simulink model "AcasXuClosedLoop_all_comp"  (Simulink 10.0)
settings: Solver=VariableStepAuto  StartTime=0.0  StopTime=20  RelTol=1e-3
libraries: neural x14040, simulink x2
(part 17 of 534)

== system AcasXuClosedLoop_all_comp/networks/Networks_m_3/AcasXu_5_3/Process Output 1 ==
  a : Inport  PortDimensions=5
      mask Display: e = 0.06;c1 = [120 230 180]/255;c2 = ([120 230 180]+0)/(256+128);c3 = ([120 230 180]+128)/(256+128);patch([0 1 1 0],[0 0 1 1],c1);patch([0 1 1 1-e 1-e 0+e],[0 0 1 1-e 0+e 0+e],c2);patch([0 0 1 1-e 0+e 0+e],[0 1 1 1-e 1-e 0+e],c3);plot([0 1 1 0 0],[0 0 1 1 0]);
  y : Outport  PortDimensions=5
      mask Display: e = 0.06;c1 = [120 230 180]/255;c2 = ([120 230 180]+0)/(256+128);c3 = ([120 230 180]+128)/(256+128);patch([0 1 1 0],[0 0 1 1],c1);patch([0 1 1 1-e 1-e 0+e],[0 0 1 1-e 0+e 0+e],c2);patch([0 0 1 1-e 0+e 0+e],[0 1 1 1-e 1-e 0+e],c3);plot([0 1 1 0 0],[0 0 1 1 0]);
  wires:
  a/1 -> y/1

== system AcasXuClosedLoop_all_comp/networks/Networks_m_4/AcasXu_1_4/Layer 1 ==
  "p{1}" : Inport  PortDimensions=5
      mask Display: e = 0.06;c1 = [120 230 180]/255;c2 = ([120 230 180]+0)/(256+128);c3 = ([120 230 180]+128)/(256+128);patch([0 1 1 0],[0 0 1 1],c1);patch([0 1 1 1-e 1-e 0+e],[0 0 1 1-e 0+e 0+e],c2);patch([0 0 1 1-e 0+e 0+e],[0 1 1 1-e 1-e 0+e],c3);plot([0 1 1 0 0],[0 0 1 1 0]);
  Delays 1 : Subsystem
      mask Display: e = 0.06;c1 = [120 150 230]/255;c2 = ([120 150 230]+0)/383;c3 = ([120 150 230]+128)/383;patch([0 1 1 0],[0 0 1 1],c1);patch([0 1 1 1-e 1-e 0+e],[0 0 1 1-e 0+e 0+e],c2);patch([0 0 1 1-e 0+e 0+e],[0 1 1 1-e 1-e 0+e],c3);plot([0 1 1 0 0],[0 0 1 1 0]);disp('0');
  "IW{1,1}" : Subsystem
      mask Display: e = 0.06;c1 = [120 150 230]/255;c2 = ([120 150 230]+0)/383;c3 = ([120 150 230]+128)/383;patch([0 1 1 0],[0 0 1 1],c1);patch([0 1 1 1-e 1-e 0+e],[0 0 1 1-e 0+e 0+e],c2);patch([0 0 1 1-e 0+e 0+e],[0 1 1 1-e 1-e 0+e],c3);plot([0 1 1 0 0],[0 0 1 1 0]);disp('W');
  "b{1}" : Constant  Value=[-0.369373000000000006881606395836570300161838531494140625;0.0031518499999999998774369291965058437199331820011138916015625;0.003017829999999999991688870437656078138388693332672119140625;0.2210229999999999972448705420902115292847156524658203125;-0.07530870000000000619166939941351301968097686767578125;-0.026249899999999999622435353785476763732731342315673828125;-0.34564899999999998403410472747054882347583770751953125;-0.1547409999999999896669322652087430469691753387451171875;-0.273818999999999979078069145543850027024745941162109375;0.17357800000000001006128513836301863193511962890625;-0.265224999999999988542498385868384502828121185302734375;0.000613303000000000046905423989329619871568866074085235595703125;-0.306727000000000027402080604588263668119907379150390625;-0.279696000000000000174082970261224545538425445556640625;0.251846000000000014296119843493215739727020263671875;0.09491080000000000349569262425575288943946361541748046875;0.07782429999999999903348424368232372216880321502685546875;-0.16648899999999999810285089552053250372409820556640625;-0.351362000000000007648992550457478500902652740478515625;-0.004800220000000000007689404668553834198974072933197021484375;0.115561999999999998056665617696125991642475128173828125;-0.255651000000000017120527218139613978564739227294921875;-0.10977699999999999957989160748184076510369777679443359375;-0.1637280000000000124149579505683504976332187652587890625;0.14482600000000001028155338644864968955516815185546875;-0.1920590000000000074908967917508562095463275909423828125;0.083848599999999995358024307279265485703945159912109375;0.041894300000000002370814655705544282682240009307861328125;-0.2147469999999999934470196194524760358035564422607421875;0.07221950000000000591260373994373367168009281158447265625;0.053900999999999997525090833505601040087640285491943359375;0.0011389699999999999997790656180995938484556972980499267578125;-0.17640800000000000924416099223890341818332672119140625;-0.0249854000000000013581580304844464990310370922088623046875;-0.281220999999999998752997498741024173796176910400390625;0.11540799999999999669864081397463451139628887176513671875;0.030775799999999998990407590326867648400366306304931640625;0.00082675499999999998122779398812554063624702394008636474609375;-0.09438370000000000092921226269027101807296276092529296875;-0.51336899999999996424548953655175864696502685546875;-0.23788500000000001310951347477384842932224273681640625;0.07809370000000000200390815052742254920303821563720703125;-0.30906000000000000138555833473219536244869232177734375;-0.1812979999999999869420008735687588341534137725830078125;0.16301799999999999624122892782907001674175262451171875;-0.1350470000000000003748112931134528480470180511474609375;0.0954322999999999976861175809972337447106838226318359375;-0.08284819999999999684536788890909519977867603302001953125;-0.1302379999999999926618698964375653304159641265869140625;0.16506399999999998851052396275917999446392059326171875]
      mask Display: e = 0.06;c1 = [120 150 230]/255;c2 = ([120 150 230]+0)/383;c3 = ([120 150 230]+128)/383;patch([0 1 1 0],[0 0 1 1],c1);patch([0 1 1 1-e 1-e 0+e],[0 0 1 1-e 0+e 0+e],c2);patch([0 0 1 1-e 0+e 0+e],[0 1 1 1-e 1-e 0+e],c3);plot([0 1 1 0 0],[0 0 1 1 0]);disp('b');
  netsum : Sum  Inputs=2  SaturateOnIntegerOverflow=on  InputSameDT=on  OutDataTypeStr=Inherit: Same as first input
      mask Display: e = 0.06;c1 = [120 150 230]/255;c2 = ([120 150 230]+0)/383;c3 = ([120 150 230]+128)/383;patch([0 1 1 0],[0 0 1 1],c1);patch([0 1 1 1-e 1-e 0+e],[0 0 1 1-e 0+e 0+e],c2);patch([0 0 1 1-e 0+e 0+e],[0 1 1 1-e 1-e 0+e],c3);plot([0 1 1 0 0],[0 0 1 1 0]);disp('+');
  poslin : neural/Transfer Functions/poslin
      settings: SourceProductName=Deep Learning Toolbox  SourceProductBaseCode=NN
  "a{1}" : Outport  InitialOutput=[0;0;0;0;0;0;0;0;0;0;0;0;0;0;0;0;0;0;0;0;0;0;0;0;0;0;0;0;0;0;0;0;0;0;0;0;0;0;0;0;0;0;0;0;0;0;0;0;0;0]
      mask Display: e = 0.06;c1 = [120 230 180]/255;c2 = ([120 230 180]+0)/(256+128);c3 = ([120 230 180]+128)/(256+128);patch([0 1 1 0],[0 0 1 1],c1);patch([0 1 1 1-e 1-e 0+e],[0 0 1 1-e 0+e 0+e],c2);patch([0 0 1 1-e 0+e 0+e],[0 1 1 1-e 1-e 0+e],c3);plot([0 1 1 0 0],[0 0 1 1 0]);
  wires:
  poslin/1 -> "a{1}"/1
  netsum/1 -> poslin/1
  "b{1}"/1 -> netsum/2
  "IW{1,1}"/1("iz{1,1}") -> netsum/1
  Delays 1/1("pd{1,1}") -> "IW{1,1}"/1("pd{1,1}")
  "p{1}"/1 -> Delays 1/1("p{1}")

== system AcasXuClosedLoop_all_comp/networks/Networks_m_4/AcasXu_1_4/Layer 2 ==
  "a{1} " : Inport  PortDimensions=50
      mask Display: e = 0.06;c1 = [120 230 180]/255;c2 = ([120 230 180]+0)/(256+128);c3 = ([120 230 180]+128)/(256+128);patch([0 1 1 0],[0 0 1 1],c1);patch([0 1 1 1-e 1-e 0+e],[0 0 1 1-e 0+e 0+e],c2);patch([0 0 1 1-e 0+e 0+e],[0 1 1 1-e 1-e 0+e],c3);plot([0 1 1 0 0],[0 0 1 1 0]);
  Delays 1 : Subsystem
      mask Display: e = 0.06;c1 = [120 150 230]/255;c2 = ([120 150 230]+0)/383;c3 = ([120 150 230]+128)/383;patch([0 1 1 0],[0 0 1 1],c1);patch([0 1 1 1-e 1-e 0+e],[0 0 1 1-e 0+e 0+e],c2);patch([0 0 1 1-e 0+e 0+e],[0 1 1 1-e 1-e 0+e],c3);plot([0 1 1 0 0],[0 0 1 1 0]);disp('0');
  "LW{2,1}" : Subsystem
      mask Display: e = 0.06;c1 = [120 150 230]/255;c2 = ([120 150 230]+0)/383;c3 = ([120 150 230]+128)/383;patch([0 1 1 0],[0 0 1 1],c1);patch([0 1 1 1-e 1-e 0+e],[0 0 1 1-e 0+e 0+e],c2);patch([0 0 1 1-e 0+e 0+e],[0 1 1 1-e 1-e 0+e],c3);plot([0 1 1 0 0],[0 0 1 1 0]);disp('W');
  "b{2}" : Constant  Value=[0.3821689999999999809432438269141130149364471435546875;0.001662480000000000034121594438829561113379895687103271484375;-0.137998000000000009546141654936945997178554534912109375;-0.2448539999999999883240064946221536956727504730224609375;-0.325384999999999979802822736019152216613292694091796875;-0.0245271999999999990027532703606993891298770904541015625;-0.1107599999999999973443465250966255553066730499267578125;-0.445537000000000016353141063518705777823925018310546875;-0.0850719000000000058481219866735045798122882843017578125;0.1055770000000000041762149294299888424575328826904296875;-0.4239129999999999842685838302713818848133087158203125;0.115090999999999998859578909105039201676845550537109375;0.0655763000000000040312642113349284045398235321044921875;-0.0162038000000000008415934615868536639027297496795654296875;0.0002406040000000000023301638396588941759546287357807159423828125;0.27527299999999998991739857956417836248874664306640625;0.25459500000000001573852159708621911704540252685546875;-0.0222078999999999991687982259236378013156354427337646484375;-0.01405660000000000069142469527605499024502933025360107421875;-0.014699099999999999666133732034722925163805484771728515625;-0.00874918000000000049831694326485376222990453243255615234375;0.2137320000000000053130833066461491398513317108154296875;0.064714400000000005253042445474420674145221710205078125;-0.034360099999999997588684408356130006723105907440185546875;0.28537000000000001254107928616576828062534332275390625;0.251249000000000000110134124042815528810024261474609375;0.145390999999999992464694287264137528836727142333984375;-0.264349000000000000643041175862890668213367462158203125;0.31018000000000001126210236179758794605731964111328125;0.218685999999999991505461593988002277910709381103515625;-0.1979849999999999943245398981161997653543949127197265625;0.203643999999999991690202705285628326237201690673828125;0.00621389999999999963209429409971562563441693782806396484375;-0.01057770000000000064355187845421824022196233272552490234375;0.25469799999999997996980027892277576029300689697265625;-0.0206958999999999997132515971998145687393844127655029296875;0.1389869999999999994333421682313201017677783966064453125;0.11972199999999999509014969589770771563053131103515625;0.457021999999999983810283765706117264926433563232421875;0.12488100000000000588951110103153041563928127288818359375;-0.004474180000000000344273498598113292246125638484954833984375;-0.22550700000000001299582663705223239958286285400390625;0.61899000000000004018119170723366551101207733154296875;-0.017492000000000000603517236186235095374286174774169921875;-0.08685249999999999914734871708787977695465087890625;0.26463300000000000711253278495860286056995391845703125;-0.0424145999999999967489117125296615995466709136962890625;0.16082099999999999173638798311003483831882476806640625;0.036690300000000002078603955624203081242740154266357421875;-0.06109900000000000053201887340037501417100429534912109375]
      mask Display: e = 0.06;c1 = [120 150 230]/255;c2 = ([120 150 230]+0)/383;c3 = ([120 150 230]+128)/383;patch([0 1 1 0],[0 0 1 1],c1);patch([0 1 1 1-e 1-e 0+e],[0 0 1 1-e 0+e 0+e],c2);patch([0 0 1 1-e 0+e 0+e],[0 1 1 1-e 1-e 0+e],c3);plot([0 1 1 0 0],[0 0 1 1 0]);disp('b');
  netsum : Sum  Inputs=2  SaturateOnIntegerOverflow=on  InputSameDT=on  OutDataTypeStr=Inherit: Same as first input
      mask Display: e = 0.06;c1 = [120 150 230]/255;c2 = ([120 150 230]+0)/383;c3 = ([120 150 230]+128)/383;patch([0 1 1 0],[0 0 1 1],c1);patch([0 1 1 1-e 1-e 0+e],[0 0 1 1-e 0+e 0+e],c2);patch([0 0 1 1-e 0+e 0+e],[0 1 1 1-e 1-e 0+e],c3);plot([0 1 1 0 0],[0 0 1 1 0]);disp('+');
  poslin : neural/Transfer Functions/poslin
      settings: SourceProductName=Deep Learning Toolbox  SourceProductBaseCode=NN
  "a{2}" : Outport  InitialOutput=[0;0;0;0;0;0;0;0;0;0;0;0;0;0;0;0;0;0;0;0;0;0;0;0;0;0;0;0;0;0;0;0;0;0;0;0;0;0;0;0;0;0;0;0;0;0;0;0;0;0]
      mask Display: e = 0.06;c1 = [120 230 180]/255;c2 = ([120 230 180]+0)/(256+128);c3 = ([120 230 180]+128)/(256+128);patch([0 1 1 0],[0 0 1 1],c1);patch([0 1 1 1-e 1-e 0+e],[0 0 1 1-e 0+e 0+e],c2);patch([0 0 1 1-e 0+e 0+e],[0 1 1 1-e 1-e 0+e],c3);plot([0 1 1 0 0],[0 0 1 1 0]);
  wires:
  poslin/1 -> "a{2}"/1
  netsum/1 -> poslin/1
  "b{2}"/1 -> netsum/2
  "LW{2,1}"/1("lz{2,1}") -> netsum/1
  Delays 1/1("pd{2,1}") -> "LW{2,1}"/1("ad{2,1}")
  "a{1} "/1 -> Delays 1/1("p{2}")

== system AcasXuClosedLoop_all_comp/networks/Networks_m_4/AcasXu_1_4/Layer 3 ==
  "a{2} " : Inport  PortDimensions=50
      mask Display: e = 0.06;c1 = [120 230 180]/255;c2 = ([120 230 180]+0)/(256+128);c3 = ([120 230 180]+128)/(256+128);patch([0 1 1 0],[0 0 1 1],c1);patch([0 1 1 1-e 1-e 0+e],[0 0 1 1-e 0+e 0+e],c2);patch([0 0 1 1-e 0+e 0+e],[0 1 1 1-e 1-e 0+e],c3);plot([0 1 1 0 0],[0 0 1 1 0]);
  Delays 1 : Subsystem
      mask Display: e = 0.06;c1 = [120 150 230]/255;c2 = ([120 150 230]+0)/383;c3 = ([120 150 230]+128)/383;patch([0 1 1 0],[0 0 1 1],c1);patch([0 1 1 1-e 1-e 0+e],[0 0 1 1-e 0+e 0+e],c2);patch([0 0 1 1-e 0+e 0+e],[0 1 1 1-e 1-e 0+e],c3);plot([0 1 1 0 0],[0 0 1 1 0]);disp('0');
  "LW{3,2}" : Subsystem
      mask Display: e = 0.06;c1 = [120 150 230]/255;c2 = ([120 150 230]+0)/383;c3 = ([120 150 230]+128)/383;patch([0 1 1 0],[0 0 1 1],c1);patch([0 1 1 1-e 1-e 0+e],[0 0 1 1-e 0+e 0+e],c2);patch([0 0 1 1-e 0+e 0+e],[0 1 1 1-e 1-e 0+e],c3);plot([0 1 1 0 0],[0 0 1 1 0]);disp('W');
  "b{3}" : Constant  Value=[0.0068958200000000004215028326370884315110743045806884765625;0.9524690000000000100754959930782206356525421142578125;0.5296499999999999541699935434735380113124847412109375;-0.6284419999999999451034682351746596395969390869140625;-0.2021139999999999881108436738941236399114131927490234375;-0.052532900000000000428901358873190474696457386016845703125;-0.00145183000000000009092893105133725839550606906414031982421875;0.152733000000000007645439836778677999973297119140625;0.12338999999999999968025576890795491635799407958984375;0.015124800000000000743671790814914857037365436553955078125;-0.018701900000000000467981209339995984919369220733642578125;0.24758299999999999752020585219725035130977630615234375;0.0617325999999999985856646844695205800235271453857421875;-0.07944500000000000172750702631674357689917087554931640625;-0.017059100000000000652011777901861933059990406036376953125;-0.02747159999999999879793932677785051055252552032470703125;-0.48753299999999999414512785733677446842193603515625;-0.039202700000000000046807002718196599744260311126708984375;0.346100000000000018740564655672642402350902557373046875;0.87589700000000003665689973786356858909130096435546875;-0.0260366999999999994275245995822842814959585666656494140625;0.07152419999999999611350887107619200833141803741455078125;-0.016593400000000001204281119271399802528321743011474609375;0.109650999999999998468780404436984099447727203369140625;0.0985657000000000060868643458888982422649860382080078125;-0.2114189999999999958202323568912106566131114959716796875;0.217096000000000011187495374542777426540851593017578125;0.02582009999999999860786914496202371083199977874755859375;0.16187099999999998711786020066938363015651702880859375;-0.00649602999999999985991649964489624835550785064697265625;-0.353837000000000012622791700778179802000522613525390625;0.434844999999999981543652438631397671997547149658203125;0.07813040000000000262492250158175011165440082550048828125;-0.2458909999999999984598986202399828471243381500244140625;-0.339401000000000008238743021138361655175685882568359375;0.376278999999999974601649910255218856036663055419921875;-0.12090599999999999958344432116064126603305339813232421875;-0.09377910000000000412256895288010127842426300048828125;-0.470905999999999991256771636471967212855815887451171875;-0.461100000000000009858780458671390078961849212646484375;0.0852379000000000053294257895686314441263675689697265625;-0.1458939999999999959090501988612231798470020294189453125;0.0291369999999999997053468092644834541715681552886962890625;-0.00843813000000000028311131217151341843418776988983154296875;-0.116458000000000005957900839348440058529376983642578125;-0.1329180000000000083648643567357794381678104400634765625;-0.97648699999999999388222704510553739964962005615234375;-0.06524770000000000569340130596174276433885097503662109375;-0.11002099999999999380140280891282600350677967071533203125;-0.305489999999999983781862056275713257491588592529296875]
      mask Display: e = 0.06;c1 = [120 150 230]/255;c2 = ([120 150 230]+0)/383;c3 = ([120 150 230]+128)/383;patch([0 1 1 0],[0 0 1 1],c1);patch([0 1 1 1-e 1-e 0+e],[0 0 1 1-e 0+e 0+e],c2);patch([0 0 1 1-e 0+e 0+e],[0 1 1 1-e 1-e 0+e],c3);plot([0 1 1 0 0],[0 0 1 1 0]);disp('b');
  netsum : Sum  Inputs=2  SaturateOnIntegerOverflow=on  InputSameDT=on  OutDataTypeStr=Inherit: Same as first input
      mask Display: e = 0.06;c1 = [120 150 230]/255;c2 = ([120 150 230]+0)/383;c3 = ([120 150 230]+128)/383;patch([0 1 1 0],[0 0 1 1],c1);patch([0 1 1 1-e 1-e 0+e],[0 0 1 1-e 0+e 0+e],c2);patch([0 0 1 1-e 0+e 0+e],[0 1 1 1-e 1-e 0+e],c3);plot([0 1 1 0 0],[0 0 1 1 0]);disp('+');
  poslin : neural/Transfer Functions/poslin
      settings: SourceProductName=Deep Learning Toolbox  SourceProductBaseCode=NN
  "a{3}" : Outport  InitialOutput=[0;0;0;0;0;0;0;0;0;0;0;0;0;0;0;0;0;0;0;0;0;0;0;0;0;0;0;0;0;0;0;0;0;0;0;0;0;0;0;0;0;0;0;0;0;0;0;0;0;0]
      mask Display: e = 0.06;c1 = [120 230 180]/255;c2 = ([120 230 180]+0)/(256+128);c3 = ([120 230 180]+128)/(256+128);patch([0 1 1 0],[0 0 1 1],c1);patch([0 1 1 1-e 1-e 0+e],[0 0 1 1-e 0+e 0+e],c2);patch([0 0 1 1-e 0+e 0+e],[0 1 1 1-e 1-e 0+e],c3);plot([0 1 1 0 0],[0 0 1 1 0]);
  wires:
  poslin/1 -> "a{3}"/1
  netsum/1 -> poslin/1
  "b{3}"/1 -> netsum/2
  "LW{3,2}"/1("lz{3,2}") -> netsum/1
  Delays 1/1("pd{3,2}") -> "LW{3,2}"/1("ad{3,2}")
  "a{2} "/1 -> Delays 1/1("p{3}")

== system AcasXuClosedLoop_all_comp/networks/Networks_m_4/AcasXu_1_4/Layer 4 ==
  "a{3} " : Inport  PortDimensions=50
      mask Display: e = 0.06;c1 = [120 230 180]/255;c2 = ([120 230 180]+0)/(256+128);c3 = ([120 230 180]+128)/(256+128);patch([0 1 1 0],[0 0 1 1],c1);patch([0 1 1 1-e 1-e 0+e],[0 0 1 1-e 0+e 0+e],c2);patch([0 0 1 1-e 0+e 0+e],[0 1 1 1-e 1-e 0+e],c3);plot([0 1 1 0 0],[0 0 1 1 0]);
  Delays 1 : Subsystem
      mask Display: e = 0.06;c1 = [120 150 230]/255;c2 = ([120 150 230]+0)/383;c3 = ([120 150 230]+128)/383;patch([0 1 1 0],[0 0 1 1],c1);patch([0 1 1 1-e 1-e 0+e],[0 0 1 1-e 0+e 0+e],c2);patch([0 0 1 1-e 0+e 0+e],[0 1 1 1-e 1-e 0+e],c3);plot([0 1 1 0 0],[0 0 1 1 0]);disp('0');
  "LW{4,3}" : Subsystem
      mask Display: e = 0.06;c1 = [120 150 230]/255;c2 = ([120 150 230]+0)/383;c3 = ([120 150 230]+128)/383;patch([0 1 1 0],[0 0 1 1],c1);patch([0 1 1 1-e 1-e 0+e],[0 0 1 1-e 0+e 0+e],c2);patch([0 0 1 1-e 0+e 0+e],[0 1 1 1-e 1-e 0+e],c3);plot([0 1 1 0 0],[0 0 1 1 0]);disp('W');
  "b{4}" : Constant  Value=[0.01553470000000000024453772340393697959370911121368408203125;-0.6310729999999999950688334138249047100543975830078125;-0.048659500000000001362909785029842169024050235748291015625;0.335438999999999987178256333208992145955562591552734375;-0.88891500000000001069366817318950779736042022705078125;-0.0137748000000000002385203146104686311446130275726318359375;-1.691289999999999960067498250282369554042816162109375;-0.0127559999999999999775734949025718378834426403045654296875;-0.00052239899999999999703759190339269480318762362003326416015625;-0.01569600000000000161737290227392804808914661407470703125;-0.251987000000000016530776747458730824291706085205078125;-0.196713999999999999968025576890795491635799407958984375;-0.5302080000000000126192389870993793010711669921875;-0.2934959999999999791242544233682565391063690185546875;-0.55378600000000000047606363295926712453365325927734375;-0.1473190000000000055013771316225756891071796417236328125;0.1564359999999999917275061989130335859954357147216796875;-0.019231100000000000971311919784056954085826873779296875;-0.1021370000000000055617732641621842049062252044677734375;-0.538108999999999948471440802677534520626068115234375;0.490462000000000009070078021977678872644901275634765625;1.2597899999999999653965687684831209480762481689453125;-1.0325800000000000533617594555835239589214324951171875;-0.6781070000000000153050905282725580036640167236328125;0.408625999999999989231724839555681683123111724853515625;0.06938740000000000185398363328204140998423099517822265625;-0.325770999999999977259079741997993551194667816162109375;-0.90760099999999999109689952092594467103481292724609375;0.751886999999999972033037920482456684112548828125;-0.142394999999999993800514630493125878274440765380859375;0.24531300000000000327560201185406185686588287353515625;0.5317840000000000344471118296496570110321044921875;-0.1820090000000000041158187968903803266584873199462890625;0.1879159999999999997033484078201581723988056182861328125;-0.411633999999999999896971303314785473048686981201171875;-0.70832300000000003592504072003066539764404296875;-0.306723000000000023401725002258899621665477752685546875;-0.25211200000000000276401124210678972303867340087890625;-0.53940200000000004809663778360118158161640167236328125;-0.5040270000000000027995383788947947323322296142578125;-0.01096920000000000013085088568232094985432922840118408203125;-0.2206249999999999877875467291232780553400516510009765625;0.33433699999999999530331251662573777139186859130859375;-0.026619099999999999706101760921228560619056224822998046875;-0.38062099999999998711786020066938363015651702880859375;-0.91636799999999996035882077194401063024997711181640625;-0.213361999999999996102673094355850480496883392333984375;0.26015800000000000036237679523765109479427337646484375;0.06854999999999999982236431605997495353221893310546875;-0.00156862999999999989421406443312889678054489195346832275390625]
      mask Display: e = 0.06;c1 = [120 150 230]/255;c2 = ([120 150 230]+0)/383;c3 = ([120 150 230]+128)/383;patch([0 1 1 0],[0 0 1 1],c1);patch([0 1 1 1-e 1-e 0+e],[0 0 1 1-e 0+e 0+e],c2);patch([0 0 1 1-e 0+e 0+e],[0 1 1 1-e 1-e 0+e],c3);plot([0 1 1 0 0],[0 0 1 1 0]);disp('b');
  netsum : Sum  Inputs=2  SaturateOnIntegerOverflow=on  InputSameDT=on  OutDataTypeStr=Inherit: Same as first input
      mask Display: e = 0.06;c1 = [120 150 230]/255;c2 = ([120 150 230]+0)/383;c3 = ([120 150 230]+128)/383;patch([0 1 1 0],[0 0 1 1],c1);patch([0 1 1 1-e 1-e 0+e],[0 0 1 1-e 0+e 0+e],c2);patch([0 0 1 1-e 0+e 0+e],[0 1 1 1-e 1-e 0+e],c3);plot([0 1 1 0 0],[0 0 1 1 0]);disp('+');
  poslin : neural/Transfer Functions/poslin
      settings: SourceProductName=Deep Learning Toolbox  SourceProductBaseCode=NN
  "a{4}" : Outport  InitialOutput=[0;0;0;0;0;0;0;0;0;0;0;0;0;0;0;0;0;0;0;0;0;0;0;0;0;0;0;0;0;0;0;0;0;0;0;0;0;0;0;0;0;0;0;0;0;0;0;0;0;0]
      mask Display: e = 0.06;c1 = [120 230 180]/255;c2 = ([120 230 180]+0)/(256+128);c3 = ([120 230 180]+128)/(256+128);patch([0 1 1 0],[0 0 1 1],c1);patch([0 1 1 1-e 1-e 0+e],[0 0 1 1-e 0+e 0+e],c2);patch([0 0 1 1-e 0+e 0+e],[0 1 1 1-e 1-e 0+e],c3);plot([0 1 1 0 0],[0 0 1 1 0]);
  wires:
  poslin/1 -> "a{4}"/1
  netsum/1 -> poslin/1
  "b{4}"/1 -> netsum/2
  "LW{4,3}"/1("lz{4,3}") -> netsum/1
  Delays 1/1("pd{4,3}") -> "LW{4,3}"/1("ad{4,3}")
  "a{3} "/1 -> Delays 1/1("p{4}")

== system AcasXuClosedLoop_all_comp/networks/Networks_m_4/AcasXu_1_4/Layer 5 ==
  "a{4} " : Inport  PortDimensions=50
      mask Display: e = 0.06;c1 = [120 230 180]/255;c2 = ([120 230 180]+0)/(256+128);c3 = ([120 230 180]+128)/(256+128);patch([0 1 1 0],[0 0 1 1],c1);patch([0 1 1 1-e 1-e 0+e],[0 0 1 1-e 0+e 0+e],c2);patch([0 0 1 1-e 0+e 0+e],[0 1 1 1-e 1-e 0+e],c3);plot([0 1 1 0 0],[0 0 1 1 0]);
  Delays 1 : Subsystem
      mask Display: e = 0.06;c1 = [120 150 230]/255;c2 = ([120 150 230]+0)/383;c3 = ([120 150 230]+128)/383;patch([0 1 1 0],[0 0 1 1],c1);patch([0 1 1 1-e 1-e 0+e],[0 0 1 1-e 0+e 0+e],c2);patch([0 0 1 1-e 0+e 0+e],[0 1 1 1-e 1-e 0+e],c3);plot([0 1 1 0 0],[0 0 1 1 0]);disp('0');
  "LW{5,4}" : Subsystem
      mask Display: e = 0.06;c1 = [120 150 230]/255;c2 = ([120 150 230]+0)/383;c3 = ([120 150 230]+128)/383;patch([0 1 1 0],[0 0 1 1],c1);patch([0 1 1 1-e 1-e 0+e],[0 0 1 1-e 0+e 0+e],c2);patch([0 0 1 1-e 0+e 0+e],[0 1 1 1-e 1-e 0+e],c3);plot([0 1 1 0 0],[0 0 1 1 0]);disp('W');
  "b{5}" : Constant  Value=[0.7618899999999999561595132036018185317516326904296875;0.5195629999999999970583530739531852304935455322265625;0.5316140000000000309654524244251661002635955810546875;-0.364360999999999990439647490347851999104022979736328125;-0.27189099999999999379696191681432537734508514404296875;-0.296509999999999995790034290621406398713588714599609375;0.2315290000000000125712773524355725385248661041259765625;0.8169969999999999732409605712746270000934600830078125;-0.2484780000000000044213521732672234065830707550048828125;0.208621000000000000884625706021324731409549713134765625;-0.07390249999999999597211086665993207134306430816650390625;-0.2057700000000000084554585555451922118663787841796875;1.43395999999999990137666827649809420108795166015625;0.8774129999999999984794385454733856022357940673828125;-0.06489799999999999735589284455272718332707881927490234375;0.75646500000000005403677505455561913549900054931640625;-0.452438999999999980072828975607990287244319915771484375;-0.4939810000000000034248159863636828958988189697265625;-0.15393699999999999050714905024506151676177978515625;0.55673600000000000864730509420041926205158233642578125;-0.003913179999999999610083012413497272063978016376495361328125;-0.10694900000000000239719355477063800208270549774169921875;0.0592174000000000033683278388707549311220645904541015625;0.075505600000000006044587053111172281205654144287109375;0.776321999999999956543206280912272632122039794921875;0.263774000000000008459011269223992712795734405517578125;0.005818679999999999685744711541701690293848514556884765625;0.330154000000000003023359340659226290881633758544921875;0.14350099999999998967581404940574429929256439208984375;0.04374569999999999847517528905882500112056732177734375;0.115844000000000002525979425627156160771846771240234375;-0.27283699999999999619149093632586300373077392578125;-0.45772299999999999098321268320432864129543304443359375;-0.42129899999999997906030557714984752237796783447265625;-0.0126625999999999995504484928687816136516630649566650390625;0.75929100000000004921929530610213987529277801513671875;-0.2051840000000000052704507425005431286990642547607421875;-0.063355400000000006155431719889747910201549530029296875;-0.245921000000000000706990022081299684941768646240234375;0.55025800000000002487610117896110750734806060791015625;-0.11560900000000000342748052162278327159583568572998046875;0.09723220000000000473594496952500776387751102447509765625;-0.0107375000000000005162537064506977912969887256622314453125;-0.04800200000000000299937852332732290960848331451416015625;-0.3592910000000000270148348135990090668201446533203125;-0.01196129999999999936333150429845773032866418361663818359375;1.0061899999999999177902054725564084947109222412109375;0.5880199999999999871391764827421866357326507568359375;-0.379718999999999973216091575523023493587970733642578125;-1.4077699999999999658228944099391810595989227294921875]
      mask Display: e = 0.06;c1 = [120 150 230]/255;c2 = ([120 150 230]+0)/383;c3 = ([120 150 230]+128)/383;patch([0 1 1 0],[0 0 1 1],c1);patch([0 1 1 1-e 1-e 0+e],[0 0 1 1-e 0+e 0+e],c2);patch([0 0 1 1-e 0+e 0+e],[0 1 1 1-e 1-e 0+e],c3);plot([0 1 1 0 0],[0 0 1 1 0]);disp('b');
  netsum : Sum  Inputs=2  SaturateOnIntegerOverflow=on  InputSameDT=on  OutDataTypeStr=Inherit: Same as first input
      mask Display: e = 0.06;c1 = [120 150 230]/255;c2 = ([120 150 230]+0)/383;c3 = ([120 150 230]+128)/383;patch([0 1 1 0],[0 0 1 1],c1);patch([0 1 1 1-e 1-e 0+e],[0 0 1 1-e 0+e 0+e],c2);patch([0 0 1 1-e 0+e 0+e],[0 1 1 1-e 1-e 0+e],c3);plot([0 1 1 0 0],[0 0 1 1 0]);disp('+');
  poslin : neural/Transfer Functions/poslin
      settings: SourceProductName=Deep Learning Toolbox  SourceProductBaseCode=NN
  "a{5}" : Outport  InitialOutput=[0;0;0;0;0;0;0;0;0;0;0;0;0;0;0;0;0;0;0;0;0;0;0;0;0;0;0;0;0;0;0;0;0;0;0;0;0;0;0;0;0;0;0;0;0;0;0;0;0;0]
      mask Display: e = 0.06;c1 = [120 230 180]/255;c2 = ([120 230 180]+0)/(256+128);c3 = ([120 230 180]+128)/(256+128);patch([0 1 1 0],[0 0 1 1],c1);patch([0 1 1 1-e 1-e 0+e],[0 0 1 1-e 0+e 0+e],c2);patch([0 0 1 1-e 0+e 0+e],[0 1 1 1-e 1-e 0+e],c3);plot([0 1 1 0 0],[0 0 1 1 0]);
  wires:
  poslin/1 -> "a{5}"/1
  netsum/1 -> poslin/1
  "b{5}"/1 -> netsum/2
  "LW{5,4}"/1("lz{5,4}") -> netsum/1
  Delays 1/1("pd{5,4}") -> "LW{5,4}"/1("ad{5,4}")
  "a{4} "/1 -> Delays 1/1("p{5}")

== system AcasXuClosedLoop_all_comp/networks/Networks_m_4/AcasXu_1_4/Layer 6 ==
  "a{5} " : Inport  PortDimensions=50
      mask Display: e = 0.06;c1 = [120 230 180]/255;c2 = ([120 230 180]+0)/(256+128);c3 = ([120 230 180]+128)/(256+128);patch([0 1 1 0],[0 0 1 1],c1);patch([0 1 1 1-e 1-e 0+e],[0 0 1 1-e 0+e 0+e],c2);patch([0 0 1 1-e 0+e 0+e],[0 1 1 1-e 1-e 0+e],c3);plot([0 1 1 0 0],[0 0 1 1 0]);
  Delays 1 : Subsystem
      mask Display: e = 0.06;c1 = [120 150 230]/255;c2 = ([120 150 230]+0)/383;c3 = ([120 150 230]+128)/383;patch([0 1 1 0],[0 0 1 1],c1);patch([0 1 1 1-e 1-e 0+e],[0 0 1 1-e 0+e 0+e],c2);patch([0 0 1 1-e 0+e 0+e],[0 1 1 1-e 1-e 0+e],c3);plot([0 1 1 0 0],[0 0 1 1 0]);disp('0');
  "LW{6,5}" : Subsystem
      mask Display: e = 0.06;c1 = [120 150 230]/255;c2 = ([120 150 230]+0)/383;c3 = ([120 150 230]+128)/383;patch([0 1 1 0],[0 0 1 1],c1);patch([0 1 1 1-e 1-e 0+e],[0 0 1 1-e 0+e 0+e],c2);patch([0 0 1 1-e 0+e 0+e],[0 1 1 1-e 1-e 0+e],c3);plot([0 1 1 0 0],[0 0 1 1 0]);disp('W');
  "b{6}" : Constant  Value=[0.0366620000000000001438849039914202876389026641845703125;0.146299000000000012366996315904543735086917877197265625;-0.27647800000000000153477230924181640148162841796875;-0.07524050000000000182343029564435710199177265167236328125;0.30331099999999999727862132203881628811359405517578125;-0.004418799999999999637834147137027684948407113552093505859375;-0.437883999999999995456079204814159311354160308837890625;-1.0918699999999998961897063054493628442287445068359375;0.1949009999999999909192638369859196245670318603515625;-0.2214299999999999879474188446693005971610546112060546875;0.09511709999999999609610057405006955377757549285888671875;0.270772999999999985920595690913614816963672637939453125;-0.0641338999999999936907357778181903995573520660400390625;0.2019640000000000046309622803164529614150524139404296875;0.36729000000000000536459765498875640332698822021484375;0.493122000000000004771294470629072748124599456787109375;-0.398608999999999991104004948283545672893524169921875;0.09974790000000000045332626541494391858577728271484375;0.303024000000000015564438626824994571506977081298828125;0.285142000000000006565414878423325717449188232421875;-0.30040400000000000435562697020941413938999176025390625;-0.691613999999999951029394651413895189762115478515625;0.005128190000000000352275986159611420589499175548553466796875;-0.0296667000000000007087219699997149291448295116424560546875;-0.60186799999999995858246393254376016557216644287109375;-0.049226300000000000611777295489446260035037994384765625;0.1932500000000000051070259132757200859487056732177734375;0.400419000000000024908075602070312015712261199951171875;0.0289287000000000016353141063518705777823925018310546875;0.33496599999999998598099182345322333276271820068359375;-0.48239900000000002222577677457593381404876708984375;0.383772000000000002017941369558684527873992919921875;-0.00797810000000000012210232824827471631579101085662841796875;-0.7975849999999999884181534071103669703006744384765625;0.292030000000000011795009413617663085460662841796875;0.08539850000000000218580709088200819678604602813720703125;-0.0246966999999999985149212733404056052677333354949951171875;0.254004999999999980797582566083292476832866668701171875;-1.08467000000000002302158463862724602222442626953125;0.461397000000000001573852159708621911704540252685546875;-0.0165199000000000005561329174952334142290055751800537109375;-1.9497500000000000941469124882132746279239654541015625;0.073854900000000001103472868635435588657855987548828125;-1.203440000000000065227823142777197062969207763671875;0.470260999999999984577669920327025465667247772216796875;4.86305000000000013627397821291964419287978671491146087646484375e-05;-0.58006999999999997452704292300040833652019500732421875;0.29770499999999999740651901447563432157039642333984375;-0.230847999999999997644550830955267883837223052978515625;0.2154600000000000126210153439387795515358448028564453125]
      mask Display: e = 0.06;c1 = [120 150 230]/255;c2 = ([120 150 230]+0)/383;c3 = ([120 150 230]+128)/383;patch([0 1 1 0],[0 0 1 1],c1);patch([0 1 1 1-e 1-e 0+e],[0 0 1 1-e 0+e 0+e],c2);patch([0 0 1 1-e 0+e 0+e],[0 1 1 1-e 1-e 0+e],c3);plot([0 1 1 0 0],[0 0 1 1 0]);disp('b');
  netsum : Sum  Inputs=2  SaturateOnIntegerOverflow=on  InputSameDT=on  OutDataTypeStr=Inherit: Same as first input
      mask Display: e = 0.06;c1 = [120 150 230]/255;c2 = ([120 150 230]+0)/383;c3 = ([120 150 230]+128)/383;patch([0 1 1 0],[0 0 1 1],c1);patch([0 1 1 1-e 1-e 0+e],[0 0 1 1-e 0+e 0+e],c2);patch([0 0 1 1-e 0+e 0+e],[0 1 1 1-e 1-e 0+e],c3);plot([0 1 1 0 0],[0 0 1 1 0]);disp('+');
  poslin : neural/Transfer Functions/poslin
      settings: SourceProductName=Deep Learning Toolbox  SourceProductBaseCode=NN
  "a{6}" : Outport  InitialOutput=[0;0;0;0;0;0;0;0;0;0;0;0;0;0;0;0;0;0;0;0;0;0;0;0;0;0;0;0;0;0;0;0;0;0;0;0;0;0;0;0;0;0;0;0;0;0;0;0;0;0]
      mask Display: e = 0.06;c1 = [120 230 180]/255;c2 = ([120 230 180]+0)/(256+128);c3 = ([120 230 180]+128)/(256+128);patch([0 1 1 0],[0 0 1 1],c1);patch([0 1 1 1-e 1-e 0+e],[0 0 1 1-e 0+e 0+e],c2);patch([0 0 1 1-e 0+e 0+e],[0 1 1 1-e 1-e 0+e],c3);plot([0 1 1 0 0],[0 0 1 1 0]);
  wires:
  poslin/1 -> "a{6}"/1
  netsum/1 -> poslin/1
  "b{6}"/1 -> netsum/2
  "LW{6,5}"/1("lz{6,5}") -> netsum/1
  Delays 1/1("pd{6,5}") -> "LW{6,5}"/1("ad{6,5}")
  "a{5} "/1 -> Delays 1/1("p{6}")

== system AcasXuClosedLoop_all_comp/networks/Networks_m_4/AcasXu_1_4/Layer 7 ==
  "a{6} " : Inport  PortDimensions=50
      mask Display: e = 0.06;c1 = [120 230 180]/255;c2 = ([120 230 180]+0)/(256+128);c3 = ([120 230 180]+128)/(256+128);patch([0 1 1 0],[0 0 1 1],c1);patch([0 1 1 1-e 1-e 0+e],[0 0 1 1-e 0+e 0+e],c2);patch([0 0 1 1-e 0+e 0+e],[0 1 1 1-e 1-e 0+e],c3);plot([0 1 1 0 0],[0 0 1 1 0]);
  Delays 1 : Subsystem
      mask Display: e = 0.06;c1 = [120 150 230]/255;c2 = ([120 150 230]+0)/383;c3 = ([120 150 230]+128)/383;patch([0 1 1 0],[0 0 1 1],c1);patch([0 1 1 1-e 1-e 0+e],[0 0 1 1-e 0+e 0+e],c2);patch([0 0 1 1-e 0+e 0+e],[0 1 1 1-e 1-e 0+e],c3);plot([0 1 1 0 0],[0 0 1 1 0]);disp('0');
  "LW{7,6}" : Subsystem
      mask Display: e = 0.06;c1 = [120 150 230]/255;c2 = ([120 150 230]+0)/383;c3 = ([120 150 230]+128)/383;patch([0 1 1 0],[0 0 1 1],c1);patch([0 1 1 1-e 1-e 0+e],[0 0 1 1-e 0+e 0+e],c2);patch([0 0 1 1-e 0+e 0+e],[0 1 1 1-e 1-e 0+e],c3);plot([0 1 1 0 0],[0 0 1 1 0]);disp('W');
  "b{7}" : Constant  Value=[-0.020512900000000000577671244172961451113224029541015625;-0.0196128999999999990844212760521259042434394359588623046875;-0.019243900000000001282440820205010822974145412445068359375;-0.019242399999999999782307469331499305553734302520751953125;-0.019344699999999999395772221078004804439842700958251953125]
      mask Display: e = 0.06;c1 = [120 150 230]/255;c2 = ([120 150 230]+0)/383;c3 = ([120 150 230]+128)/383;patch([0 1 1 0],[0 0 1 1],c1);patch([0 1 1 1-e 1-e 0+e],[0 0 1 1-e 0+e 0+e],c2);patch([0 0 1 1-e 0+e 0+e],[0 1 1 1-e 1-e 0+e],c3);plot([0 1 1 0 0],[0 0 1 1 0]);disp('b');
  netsum : Sum  Inputs=2  SaturateOnIntegerOverflow=on  InputSameDT=on  OutDataTypeStr=Inherit: Same as first input
      mask Display: e = 0.06;c1 = [120 150 230]/255;c2 = ([120 150 230]+0)/383;c3 = ([120 150 230]+128)/383;patch([0 1 1 0],[0 0 1 1],c1);patch([0 1 1 1-e 1-e 0+e],[0 0 1 1-e 0+e 0+e],c2);patch([0 0 1 1-e 0+e 0+e],[0 1 1 1-e 1-e 0+e],c3);plot([0 1 1 0 0],[0 0 1 1 0]);disp('+');
  purelin : neural/Transfer Functions/purelin
      settings: SourceProductName=Deep Learning Toolbox
  "a{7}" : Outport  InitialOutput=[0;0;0;0;0]
      mask Display: e = 0.06;c1 = [120 230 180]/255;c2 = ([120 230 180]+0)/(256+128);c3 = ([120 230 180]+128)/(256+128);patch([0 1 1 0],[0 0 1 1],c1);patch([0 1 1 1-e 1-e 0+e],[0 0 1 1-e 0+e 0+e],c2);patch([0 0 1 1-e 0+e 0+e],[0 1 1 1-e 1-e 0+e],c3);plot([0 1 1 0 0],[0 0 1 1 0]);
  wires:
  purelin/1 -> "a{7}"/1
  netsum/1 -> purelin/1
  "b{7}"/1 -> netsum/2
  "LW{7,6}"/1("lz{7,6}") -> netsum/1
  Delays 1/1("pd{7,6}") -> "LW{7,6}"/1("ad{7,6}")
  "a{6} "/1 -> Delays 1/1("p{7}")

== system AcasXuClosedLoop_all_comp/networks/Networks_m_4/AcasXu_1_4/Process Input 1 ==
  x : Inport  PortDimensions=5
      mask Display: e = 0.06;c1 = [120 230 180]/255;c2 = ([120 230 180]+0)/(256+128);c3 = ([120 230 180]+128)/(256+128);patch([0 1 1 0],[0 0 1 1],c1);patch([0 1 1 1-e 1-e 0+e],[0 0 1 1-e 0+e 0+e],c2);patch([0 0 1 1-e 0+e 0+e],[0 1 1 1-e 1-e 0+e],c3);plot([0 1 1 0 0],[0 0 1 1 0]);
  p : Outport  PortDimensions=5
      mask Display: e = 0.06;c1 = [120 230 180]/255;c2 = ([120 230 180]+0)/(256+128);c3 = ([120 230 180]+128)/(256+128);patch([0 1 1 0],[0 0 1 1],c1);patch([0 1 1 1-e 1-e 0+e],[0 0 1 1-e 0+e 0+e],c2);patch([0 0 1 1-e 0+e 0+e],[0 1 1 1-e 1-e 0+e],c3);plot([0 1 1 0 0],[0 0 1 1 0]);
  wires:
  x/1 -> p/1

== system AcasXuClosedLoop_all_comp/networks/Networks_m_4/AcasXu_1_4/Process Output 1 ==
  a : Inport  PortDimensions=5
      mask Display: e = 0.06;c1 = [120 230 180]/255;c2 = ([120 230 180]+0)/(256+128);c3 = ([120 230 180]+128)/(256+128);patch([0 1 1 0],[0 0 1 1],c1);patch([0 1 1 1-e 1-e 0+e],[0 0 1 1-e 0+e 0+e],c2);patch([0 0 1 1-e 0+e 0+e],[0 1 1 1-e 1-e 0+e],c3);plot([0 1 1 0 0],[0 0 1 1 0]);
  y : Outport  PortDimensions=5
      mask Display: e = 0.06;c1 = [120 230 180]/255;c2 = ([120 230 180]+0)/(256+128);c3 = ([120 230 180]+128)/(256+128);patch([0 1 1 0],[0 0 1 1],c1);patch([0 1 1 1-e 1-e 0+e],[0 0 1 1-e 0+e 0+e],c2);patch([0 0 1 1-e 0+e 0+e],[0 1 1 1-e 1-e 0+e],c3);plot([0 1 1 0 0],[0 0 1 1 0]);
  wires:
  a/1 -> y/1

== system AcasXuClosedLoop_all_comp/networks/Networks_m_4/AcasXu_2_4/Layer 1 ==
  "p{1}" : Inport  PortDimensions=5
      mask Display: e = 0.06;c1 = [120 230 180]/255;c2 = ([120 230 180]+0)/(256+128);c3 = ([120 230 180]+128)/(256+128);patch([0 1 1 0],[0 0 1 1],c1);patch([0 1 1 1-e 1-e 0+e],[0 0 1 1-e 0+e 0+e],c2);patch([0 0 1 1-e 0+e 0+e],[0 1 1 1-e 1-e 0+e],c3);plot([0 1 1 0 0],[0 0 1 1 0]);
  Delays 1 : Subsystem
      mask Display: e = 0.06;c1 = [120 150 230]/255;c2 = ([120 150 230]+0)/383;c3 = ([120 150 230]+128)/383;patch([0 1 1 0],[0 0 1 1],c1);patch([0 1 1 1-e 1-e 0+e],[0 0 1 1-e 0+e 0+e],c2);patch([0 0 1 1-e 0+e 0+e],[0 1 1 1-e 1-e 0+e],c3);plot([0 1 1 0 0],[0 0 1 1 0]);disp('0');
  "IW{1,1}" : Subsystem
      mask Display: e = 0.06;c1 = [120 150 230]/255;c2 = ([120 150 230]+0)/383;c3 = ([120 150 230]+128)/383;patch([0 1 1 0],[0 0 1 1],c1);patch([0 1 1 1-e 1-e 0+e],[0 0 1 1-e 0+e 0+e],c2);patch([0 0 1 1-e 0+e 0+e],[0 1 1 1-e 1-e 0+e],c3);plot([0 1 1 0 0],[0 0 1 1 0]);disp('W');
  "b{1}" : Constant  Value=[-0.0193207999999999990858867704446311108767986297607421875;-0.108101000000000002643218977027572691440582275390625;0.0166607999999999999152233698396230465732514858245849609375;0.161544999999999994155785998373175971210002899169921875;-0.1069299999999999972732922515206155367195606231689453125;0.289727000000000012303047469686134718358516693115234375;0.6571219999999999838991016076761297881603240966796875;-0.1492650000000000087840845708342385478317737579345703125;-0.1428490000000000037516656448133289813995361328125;-0.6060410000000000518838305652025155723094940185546875;-0.50861699999999998578203985744039528071880340576171875;-0.31538699999999997292121634018258191645145416259765625;0.056099799999999998278976676147067337296903133392333984375;-0.0207993999999999991390442488636836060322821140289306640625;0.04958769999999999844586540120872086845338344573974609375;0.35922799999999999176480969254043884575366973876953125;-0.1349490000000000133884014985596877522766590118408203125;-0.04803000000000000324629212400395772419869899749755859375;0.1137350000000000027622348852673894725739955902099609375;0.055233100000000000251443310617105453275144100189208984375;0.1875570000000000014939161019356106407940387725830078125;-0.56689299999999998025401737322681583464145660400390625;0.05597169999999999923101512422363157384097576141357421875;-0.4592439999999999855617716093547642230987548828125;0.145340999999999997971400489404913969337940216064453125;-0.019313799999999999024158370275472407229244709014892578125;0.06927319999999999311501852616856922395527362823486328125;0.095305699999999993199395476040081121027469635009765625;0.10167099999999999748734325066834571771323680877685546875;0.11587899999999999589572752256572130136191844940185546875;-0.457239000000000006540545882671722210943698883056640625;-0.41383700000000001040234565152786672115325927734375;-0.332726000000000021739055000580265186727046966552734375;-0.05297169999999999656647986512325587682425975799560546875;0.060874699999999996868904617031148518435657024383544921875;0.38063700000000000311928260998683981597423553466796875;0.23968800000000001215738620885531418025493621826171875;0.03134030000000000149018575257287011481821537017822265625;0.244431000000000009375611398354521952569484710693359375;0.1687279999999999891002744334400631487369537353515625;-0.1273950000000000082334139506201609037816524505615234375;0.047209099999999996899457954668832826428115367889404296875;0.09330660000000000342534889341550297103822231292724609375;-0.205633000000000010221157253909041173756122589111328125;-0.020451099999999999834443542567896656692028045654296875;-0.328546999999999977948306195685290731489658355712890625;0.067123299999999996856558936997316777706146240234375;-0.274268000000000011784351272581261582672595977783203125;-0.53935400000000000009237055564881302416324615478515625;-0.60429100000000002257394271509838290512561798095703125]
      mask Display: e = 0.06;c1 = [120 150 230]/255;c2 = ([120 150 230]+0)/383;c3 = ([120 150 230]+128)/383;patch([0 1 1 0],[0 0 1 1],c1);patch([0 1 1 1-e 1-e 0+e],[0 0 1 1-e 0+e 0+e],c2);patch([0 0 1 1-e 0+e 0+e],[0 1 1 1-e 1-e 0+e],c3);plot([0 1 1 0 0],[0 0 1 1 0]);disp('b');
  netsum : Sum  Inputs=2  SaturateOnIntegerOverflow=on  InputSameDT=on  OutDataTypeStr=Inherit: Same as first input
      mask Display: e = 0.06;c1 = [120 150 230]/255;c2 = ([120 150 230]+0)/383;c3 = ([120 150 230]+128)/383;patch([0 1 1 0],[0 0 1 1],c1);patch([0 1 1 1-e 1-e 0+e],[0 0 1 1-e 0+e 0+e],c2);patch([0 0 1 1-e 0+e 0+e],[0 1 1 1-e 1-e 0+e],c3);plot([0 1 1 0 0],[0 0 1 1 0]);disp('+');
  poslin : neural/Transfer Functions/poslin
      settings: SourceProductName=Deep Learning Toolbox  SourceProductBaseCode=NN
  "a{1}" : Outport  InitialOutput=[0;0;0;0;0;0;0;0;0;0;0;0;0;0;0;0;0;0;0;0;0;0;0;0;0;0;0;0;0;0;0;0;0;0;0;0;0;0;0;0;0;0;0;0;0;0;0;0;0;0]
      mask Display: e = 0.06;c1 = [120 230 180]/255;c2 = ([120 230 180]+0)/(256+128);c3 = ([120 230 180]+128)/(256+128);patch([0 1 1 0],[0 0 1 1],c1);patch([0 1 1 1-e 1-e 0+e],[0 0 1 1-e 0+e 0+e],c2);patch([0 0 1 1-e 0+e 0+e],[0 1 1 1-e 1-e 0+e],c3);plot([0 1 1 0 0],[0 0 1 1 0]);
  wires:
  poslin/1 -> "a{1}"/1
  netsum/1 -> poslin/1
  "b{1}"/1 -> netsum/2
  "IW{1,1}"/1("iz{1,1}") -> netsum/1
  Delays 1/1("pd{1,1}") -> "IW{1,1}"/1("pd{1,1}")
  "p{1}"/1 -> Delays 1/1("p{1}")

== system AcasXuClosedLoop_all_comp/networks/Networks_m_4/AcasXu_2_4/Layer 2 ==
  "a{1} " : Inport  PortDimensions=50
      mask Display: e = 0.06;c1 = [120 230 180]/255;c2 = ([120 230 180]+0)/(256+128);c3 = ([120 230 180]+128)/(256+128);patch([0 1 1 0],[0 0 1 1],c1);patch([0 1 1 1-e 1-e 0+e],[0 0 1 1-e 0+e 0+e],c2);patch([0 0 1 1-e 0+e 0+e],[0 1 1 1-e 1-e 0+e],c3);plot([0 1 1 0 0],[0 0 1 1 0]);
  Delays 1 : Subsystem
      mask Display: e = 0.06;c1 = [120 150 230]/255;c2 = ([120 150 230]+0)/383;c3 = ([120 150 230]+128)/383;patch([0 1 1 0],[0 0 1 1],c1);patch([0 1 1 1-e 1-e 0+e],[0 0 1 1-e 0+e 0+e],c2);patch([0 0 1 1-e 0+e 0+e],[0 1 1 1-e 1-e 0+e],c3);plot([0 1 1 0 0],[0 0 1 1 0]);disp('0');
  "LW{2,1}" : Subsystem
      mask Display: e = 0.06;c1 = [120 150 230]/255;c2 = ([120 150 230]+0)/383;c3 = ([120 150 230]+128)/383;patch([0 1 1 0],[0 0 1 1],c1);patch([0 1 1 1-e 1-e 0+e],[0 0 1 1-e 0+e 0+e],c2);patch([0 0 1 1-e 0+e 0+e],[0 1 1 1-e 1-e 0+e],c3);plot([0 1 1 0 0],[0 0 1 1 0]);disp('W');
  "b{2}" : Constant  Value=[-0.8007619999999999738093947598827071487903594970703125;-0.8575829999999999841975295566953718662261962890625;0.65917499999999995541344333105371333658695220947265625;0.08087729999999999919158000238894601352512836456298828125;-0.177806999999999992834176509859389625489711761474609375;-0.006390289999999999859259247614318155683577060699462890625;-0.00580665999999999994651833645775695913471281528472900390625;0.10438300000000000355981910615810193121433258056640625;-0.005509499999999999640676318080068085691891610622406005859375;-0.83148999999999995136334973722114227712154388427734375;-0.86546299999999998231459130693110637366771697998046875;-0.271255000000000023874235921539366245269775390625;-0.28119499999999997275068608360015787184238433837890625;0.2635899999999999909761072558467276394367218017578125;-0.031772799999999996878319308279969845898449420928955078125;0.576041000000000025238477974198758602142333984375;-0.0225719999999999983153475824337874655611813068389892578125;0.12814300000000000689936996423057280480861663818359375;-0.161534000000000010910383707596338354051113128662109375;-0.118344000000000004746425474877469241619110107421875;0.83149200000000000887467876964365132153034210205078125;0.71636299999999997201172163840965367853641510009765625;-0.0194863000000000015365930750022016582079231739044189453125;-0.135066999999999992621013689131359569728374481201171875;-0.0435421000000000002483346861481550149619579315185546875;-0.2086929999999999896242997010631370358169078826904296875;-0.01187359999999999977948750284895140794105827808380126953125;0.060415200000000002233946361229754984378814697265625;-0.00902783000000000057649884865895728580653667449951171875;-0.1203889999999999960156316092252382077276706695556640625;0.0035615299999999998174782245285996395978145301342010498046875;0.77551800000000004065014991283533163368701934814453125;0.74551000000000000600408611717284657061100006103515625;-0.450915000000000010249578963339445181190967559814453125;-0.72735099999999996978061744812293909490108489990234375;-0.8860829999999999539994632868911139667034149169921875;0.19703999999999999293009977918700315058231353759765625;-0.408814000000000010714984455262310802936553955078125;-0.048922000000000000208277839419679366983473300933837890625;-0.060755599999999999882849266441553481854498386383056640625;0.2156470000000000053486104434341541491448879241943359375;-0.0175498000000000008158362874155500321649014949798583984375;-0.3286160000000000191988647202379070222377777099609375;0.61246699999999998365041165016009472310543060302734375;-0.58259300000000002750510930127347819507122039794921875;-0.0145021000000000004070965786695524002425372600555419921875;-0.404486999999999985444532057954347692430019378662109375;-1.0746599999999999486277602045447565615177154541015625;-0.625059999999999948983031572424806654453277587890625;-0.1860429999999999861604038642326486296951770782470703125]
      mask Display: e = 0.06;c1 = [120 150 230]/255;c2 = ([120 150 230]+0)/383;c3 = ([120 150 230]+128)/383;patch([0 1 1 0],[0 0 1 1],c1);patch([0 1 1 1-e 1-e 0+e],[0 0 1 1-e 0+e 0+e],c2);patch([0 0 1 1-e 0+e 0+e],[0 1 1 1-e 1-e 0+e],c3);plot([0 1 1 0 0],[0 0 1 1 0]);disp('b');
  netsum : Sum  Inputs=2  SaturateOnIntegerOverflow=on  InputSameDT=on  OutDataTypeStr=Inherit: Same as first input
      mask Display: e = 0.06;c1 = [120 150 230]/255;c2 = ([120 150 230]+0)/383;c3 = ([120 150 230]+128)/383;patch([0 1 1 0],[0 0 1 1],c1);patch([0 1 1 1-e 1-e 0+e],[0 0 1 1-e 0+e 0+e],c2);patch([0 0 1 1-e 0+e 0+e],[0 1 1 1-e 1-e 0+e],c3);plot([0 1 1 0 0],[0 0 1 1 0]);disp('+');
  poslin : neural/Transfer Functions/poslin
      settings: SourceProductName=Deep Learning Toolbox  SourceProductBaseCode=NN
  "a{2}" : Outport  InitialOutput=[0;0;0;0;0;0;0;0;0;0;0;0;0;0;0;0;0;0;0;0;0;0;0;0;0;0;0;0;0;0;0;0;0;0;0;0;0;0;0;0;0;0;0;0;0;0;0;0;0;0]
      mask Display: e = 0.06;c1 = [120 230 180]/255;c2 = ([120 230 180]+0)/(256+128);c3 = ([120 230 180]+128)/(256+128);patch([0 1 1 0],[0 0 1 1],c1);patch([0 1 1 1-e 1-e 0+e],[0 0 1 1-e 0+e 0+e],c2);patch([0 0 1 1-e 0+e 0+e],[0 1 1 1-e 1-e 0+e],c3);plot([0 1 1 0 0],[0 0 1 1 0]);
  wires:
  poslin/1 -> "a{2}"/1
  netsum/1 -> poslin/1
  "b{2}"/1 -> netsum/2
  "LW{2,1}"/1("lz{2,1}") -> netsum/1
  Delays 1/1("pd{2,1}") -> "LW{2,1}"/1("ad{2,1}")
  "a{1} "/1 -> Delays 1/1("p{2}")
